simulink model "TosAeb"  (R2021a)
settings: Solver=FixedStepDiscrete  StartTime=0.0  StopTime=inf  FixedStep=0.01  RelTol=1e-3
undefined symbols (not defined in the file or in its folder): ACCModSts, AccSts1, AEB_ObjectMovingOrientation, AEB_ObjectMovingState, CN_BTCActive, CN_CurrentGearR, CN_CurrentGearR_DHT, CN_PTCActive, CN_VDCActive, CN_VSC_EspOff, CN_VSC_FCW_AEB_Error, CN_VSC_FCW_AEB_NoDispay, CN_VSC_FCW_AEB_PerfmDgdtn, CN_VSC_FCW_AEB_TempUnAvlbl, CN_VSC_VehStandstill, CNaccelAvoidMax, CNaccelAvoidMin, CNbrakePedalPress, CNcomfortStrLeftAccelMax, CNcomfortStrLeftAccelMin, CNcomfortStrRightAccelMax, CNcomfortStrRightAccelMin, CNDrvDoorOpen, CNDrvModeEco, CNDrvModeNorm, CNDrvModeOther, CNDrvModeSport, CNDrvSBRreminder, CNNull, CNobjInvalidID, CNPassDoorOpen, CNPassSBRreminder, CNPEBSAccelNegInf, CNPEBSAccelNull, CNPEBSTimeInf, CNPEBSTimeNull, CNSTOPMAX, CNSTOPNULL, CNsubjWidth, CNSwtOFF, CNSwtON, CNTTCMAX, CNTTCNULL, CNVehConfigAEBCycNone, CNVehConfigAEBNone, CNVehConfigAEBPedNone, CNVehConfigFCWNone, CNVehConfigJANone, CollisionSeverity, FALSE, FCWSnvty, JACycObjCalcInfoDefaultParams, JAPedObjCalcInfoDefaultParams, JAVehObjCalcInfoDefaultParams, latCycObjCalcInfoDefaultParams, latPedObjCalcInfoDefaultParams, longObjCalcInfoDefaultParams, ModSts, movingStatus, ObjectType ... (+1112)
libraries: simulink x3031, AEBSFunctionLib x50, Ta_Library x33, AEBSStateMachineLib x23, Mylibrary x12, simulink_extras x7, ObjSelect_lib x3, dspstat3 x2
(part 78 of 102)

== system TosAeb/FcwAebJa_Step_sys/ALG_AEBSystem/ALG_AEBController/AEB_LatObjInfoCalcApp/LatPedObjCalc/LatPedCollisonAssessInfo/LatPedCollisonAssessInfo/LatPedLongDistCondCalc/PedDistBrakeFullCalc/DistPhase3Calc/tPhase3Calc/tVelDeltaCalc ==
  velDelta : Inport  OutDataTypeStr=single
  subjLongAccelFilt : Inport  Port=2  OutDataTypeStr=single
  tJerkWork : Inport  Port=3  OutDataTypeStr=single
  Kjerk : Inport  Port=4  OutDataTypeStr=single
  accelMax : Inport  Port=5  OutDataTypeStr=single
  Add : Sum  Inputs=+--  OutDataTypeStr=single
  Constant : Constant  Value=0.5  OutDataTypeStr=single
  Divide : Product  Inputs=*/  OutDataTypeStr=single
  Product : Product  Inputs=4  OutDataTypeStr=single
  Product1 : Product  OutDataTypeStr=single
  tVelDelta : Outport
  wires:
  Divide/1 -> tVelDelta/1
  accelMax/1 -> Divide/2
  Kjerk/1 -> Product/3
  velDelta/1 -> Add/1
  Add/1 -> Divide/1
  Product1/1 -> Add/2
  Constant/1 -> Product/4
  tJerkWork/1 -> Product/2, Product/1, Product1/2
  Product/1 -> Add/3
  subjLongAccelFilt/1 -> Product1/1

== system TosAeb/FcwAebJa_Step_sys/ALG_AEBSystem/ALG_AEBController/AEB_LatObjInfoCalcApp/LatPedObjCalc/LatPedObjCalc/latPedObjInfoCalc/ObjColiisionRiskProbCalc/CollisionRiskProbCalc/ObjReginCorrCalc/AccelXYMaxMinCorrCalc/AccelXMaxMinCorrCalc ==
  vxAbsTargetCorr : Inport
  AccelMaxX : Inport  Port=2  OutDataTypeStr=single
  AccelMinX : Inport  Port=3  OutDataTypeStr=single
  Bus Creator : BusCreator  Inputs=2
  Bus Creator1 : BusCreator  Inputs=2
  Bus Creator2 : BusCreator  Inputs=2
  If : If  IfExpression=u1 < 0  ElseIfExpressions=u1 > 0
  Merge : Merge  Inputs=3
  ObjDwn2UpMoving : Subsystem  TreatAsAtomicUnit=on
  ObjStanding : Subsystem  TreatAsAtomicUnit=on
  ObjUp2DwnMoving : Subsystem  TreatAsAtomicUnit=on
  AccelXMaxMinCorr : Outport
  wires:
  [AccelMinX] -> ObjDwn2UpMoving/2(AccelMinX)
  vxAbsTargetCorr/1 -> If/1
  If/1 -> ObjUp2DwnMoving/Action
  If/2 -> [ObjDwn2Up]
  If/3 -> [ObjStand]
  [ObjDwn2Up] -> ObjDwn2UpMoving/Action
  [ObjStand] -> ObjStanding/Action
  AccelMinX/1 -> ObjUp2DwnMoving/2(AccelMinX), [AccelMinX]
  AccelMaxX/1 -> ObjUp2DwnMoving/1(AccelMaxX), ObjDwn2UpMoving/1(AccelMaxX)
  ObjUp2DwnMoving/1(AccelXMaxCorr) -> Bus Creator/1  "AccelXMaxCorr"
  ObjUp2DwnMoving/2(AccelXMinCorr) -> Bus Creator/2  "AccelXMinCorr"
  ObjDwn2UpMoving/1(AccelXMaxCorr) -> Bus Creator1/1  "AccelXMaxCorr"
  ObjDwn2UpMoving/2(AccelXMinCorr) -> Bus Creator1/2  "AccelXMinCorr"
  ObjStanding/1(AccelXMaxCorr) -> Bus Creator2/1  "AccelXMaxCorr"
  ObjStanding/2(AccelXMinCorr) -> Bus Creator2/2  "AccelXMinCorr"
  Bus Creator1/1 -> Merge/2
  Bus Creator/1 -> Merge/1
  Bus Creator2/1 -> Merge/3
  Merge/1 -> AccelXMaxMinCorr/1  "AccelXMaxMinCorr"

== system TosAeb/FcwAebJa_Step_sys/ALG_AEBSystem/ALG_AEBController/AEB_LatObjInfoCalcApp/LatPedObjCalc/LatPedObjCalc/latPedObjInfoCalc/ObjColiisionRiskProbCalc/CollisionRiskProbCalc/ObjReginCorrCalc/AccelXYMaxMinCorrCalc/AccelXYMaxMinCalc ==
  maxAccelDecel : Inport
  sincosCoef : Inport  Port=2
  Abs{1..4} (4 alike) : Abs
  Bus Creator : BusCreator  Inputs=2
  Bus Selector : BusSelector  OutputSignals=maxAccelDecel.maxAccel
  Bus Selector1 : BusSelector  OutputSignals=sincosCoef.cosCoef
  Bus Selector2 : BusSelector  OutputSignals=maxAccelDecel.maxDecel
  Bus Selector3 : BusSelector  OutputSignals=sincosCoef.cosCoef
  Bus Selector4 : BusSelector  OutputSignals=maxAccelDecel.maxAccel
  Bus Selector5 : BusSelector  OutputSignals=sincosCoef.sinCoef
  Bus Selector6 : BusSelector  OutputSignals=maxAccelDecel.maxDecel
  Bus Selector7 : BusSelector  OutputSignals=sincosCoef.sinCoef
  Product{2,4..6} (4 alike) : Product  OutDataTypeStr=single
  AccelXMax : Outport
  AccelXMin : Outport  Port=2
  AccelYMax : Outport  Port=3
  AccelYMin : Outport  Port=4
  wires:
  maxAccelDecel/1 -> Bus Creator/1
  sincosCoef/1 -> Bus Creator/2
  Bus Selector5/1 -> Abs1/1  "<sinCoef>"
  Bus Selector1/1 -> Abs4/1  "<cosCoef>"
  Bus Selector3/1 -> Abs3/1  "<cosCoef>"
  Bus Selector/1 -> Product2/1  "<maxAccel>"
  Product6/1 -> AccelYMin/1  "AccelYMin"
  Bus Selector4/1 -> Product5/1  "<maxAccel>"
  Bus Selector2/1 -> Product4/1  "<maxDecel>"
  Product4/1 -> AccelXMin/1  "AccelXMin"
  Bus Creator/1 -> Bus Selector7/1, Bus Selector6/1, Bus Selector5/1, Bus Selector4/1, Bus Selector3/1, Bus Selector2/1, Bus Selector1/1, Bus Selector/1
  Bus Selector7/1 -> Abs2/1  "<sinCoef>"
  Bus Selector6/1 -> Product6/1  "<maxDecel>"
  Product5/1 -> AccelYMax/1  "AccelYMax"
  Product2/1 -> AccelXMax/1  "AccelXMax"
  Abs2/1 -> Product6/2
  Abs1/1 -> Product5/2
  Abs3/1 -> Product4/2
  Abs4/1 -> Product2/2

== system TosAeb/FcwAebJa_Step_sys/ALG_AEBSystem/ALG_AEBController/AEB_LatObjInfoCalcApp/LatPedObjCalc/LatPedObjCalc/latPedObjInfoCalc/ObjColiisionRiskProbCalc/CollisionRiskProbCalc/ObjReginCorrCalc/AccelXYMaxMinCorrCalc/AccelYMaxMinCorrCalc ==
  vyAbsTargetCorr : Inport
  AccelMaxY : Inport  Port=2  OutDataTypeStr=single
  AccelMinY : Inport  Port=3  OutDataTypeStr=single
  Bus Creator : BusCreator  Inputs=2
  Bus Creator1 : BusCreator  Inputs=2
  Bus Creator2 : BusCreator  Inputs=2
  If : If  IfExpression=u1 < 0  ElseIfExpressions=u1 > 0
  Merge : Merge  Inputs=3
  ObjLeft2RightMoving : Subsystem  TreatAsAtomicUnit=on
  ObjRight2LeftMoving : Subsystem  TreatAsAtomicUnit=on
  ObjStanding : Subsystem  TreatAsAtomicUnit=on
  AccelYMaxMinCorr : Outport
  wires:
  Merge/1 -> AccelYMaxMinCorr/1  "AccelYMaxMinCorr"
  Bus Creator2/1 -> Merge/3
  Bus Creator/1 -> Merge/1
  Bus Creator1/1 -> Merge/2
  ObjStanding/2(AccelYMinCorr) -> Bus Creator2/2  "AccelYMinCorr"
  ObjStanding/1(AccelYMaxCorr) -> Bus Creator2/1  "AccelYMaxCorr"
  ObjRight2LeftMoving/2(AccelYMinCorr) -> Bus Creator1/2  "AccelYMinCorr"
  ObjRight2LeftMoving/1(AccelYMaxCorr) -> Bus Creator1/1  "AccelYMaxCorr"
  ObjLeft2RightMoving/2(AccelYMinCorr) -> Bus Creator/2  "AccelYMinCorr"
  ObjLeft2RightMoving/1(AccelYMaxCorr) -> Bus Creator/1  "AccelYMaxCorr"
  AccelMaxY/1 -> ObjRight2LeftMoving/1(AccelYMax), ObjLeft2RightMoving/1(AccelYMax)
  AccelMinY/1 -> [AccelMinX], ObjLeft2RightMoving/2(AccelYMin)
  [ObjStand] -> ObjStanding/Action
  [ObjRight2Left] -> ObjRight2LeftMoving/Action
  If/3 -> [ObjStand]
  If/2 -> [ObjRight2Left]
  If/1 -> ObjLeft2RightMoving/Action
  vyAbsTargetCorr/1 -> If/1
  [AccelMinX] -> ObjRight2LeftMoving/2(AccelYMin)

== system TosAeb/FcwAebJa_Step_sys/ALG_AEBSystem/ALG_AEBController/AEB_LatObjInfoCalcApp/LatPedObjCalc/LatPedObjCalc/latPedObjInfoCalc/ObjColiisionRiskProbCalc/CollisionRiskProbCalc/ObjReginCorrCalc/AccelXYMaxMinCorrCalc/sincosCoefCalc ==
  angle : Inport
  Bus Creator : BusCreator  Inputs=2
  Sincos : Trigonometry  Operator=sincos
  sincosCoef : Outport
  wires:
  Sincos/1 -> Bus Creator/1  "sinCoef"
  angle/1 -> Sincos/1
  Sincos/2 -> Bus Creator/2  "cosCoef"
  Bus Creator/1 -> sincosCoef/1  "sincosCoef"

== system TosAeb/FcwAebJa_Step_sys/ALG_AEBSystem/ALG_AEBController/AEB_LatObjInfoCalcApp/LatPedObjCalc/LatPedObjCalc/latPedObjInfoCalc/ObjColiisionRiskProbCalc/CollisionRiskProbCalc/ObjReginCorrCalc/AccelXYMaxMinCorrCalc/vXYAngleCalc ==
  note: vy
      | vxAngle = atan(--------)
      | 				vx
  velAbsTargetCorr : Inport
  Bus Selector : BusSelector  OutputSignals=vyAbsObjCorr,vxAbsObjCorr
  Trigonometric Function : Trigonometry  Operator=atan2
  vXYAngle : Outport
  wires:
  velAbsTargetCorr/1 -> Bus Selector/1
  Trigonometric Function/1 -> vXYAngle/1
  Bus Selector/1 -> Trigonometric Function/1  "<vyAbsObjCorr>"
  Bus Selector/2 -> Trigonometric Function/2  "<vxAbsObjCorr>"

== system TosAeb/FcwAebJa_Step_sys/ALG_AEBSystem/ALG_AEBController/AEB_LatObjInfoCalcApp/LatPedObjCalc/LatPedObjCalc/latPedObjInfoCalc/ObjColiisionRiskProbCalc/CollisionRiskProbCalc/ObjReginCorrCalc/PedMaxAccelCalc/MaxAccelJoggingMode ==
  tPredTime : Inport  OutDataTypeStr=single
  Action Port : ActionPort  ActionPortLabel=case [ 2 ]:
  Bus Creator : BusCreator  Inputs=2
  MaxAccelJoggingModeLookupTable : Lookup_n-D  NumberOfTableDimensions=1  BreakpointsForDimension1=P_AEB_LatPedCA_CollisionRiskProbJoggingStsMaxAccelX  BeginIndexSearchUsingPreviousIndexResult=on  Table=P_AEB_LatPedCA_CollisionRiskProbJoggingStsMaxAccelY  ExtrapMethod=Clip  InternalRulePriority=Speed  OutDataTypeStr=single  RndMeth=Simplest  InputSameDT=on
  MaxDecelJoggingModeLookupTable1 : Lookup_n-D  NumberOfTableDimensions=1  BreakpointsForDimension1=P_AEB_LatPedCA_CollisionRiskProbJoggingStsMaxDecelX  BeginIndexSearchUsingPreviousIndexResult=on  Table=P_AEB_LatPedCA_CollisionRiskProbJoggingStsMaxDecelY  ExtrapMethod=Clip  InternalRulePriority=Speed  OutDataTypeStr=single  RndMeth=Simplest  InputSameDT=on
  maxAccelDecel : Outport
  wires:
  tPredTime/1 -> MaxDecelJoggingModeLookupTable1/1, MaxAccelJoggingModeLookupTable/1
  MaxAccelJoggingModeLookupTable/1 -> Bus Creator/1  "maxAccel"
  Bus Creator/1 -> maxAccelDecel/1
  MaxDecelJoggingModeLookupTable1/1 -> Bus Creator/2  "maxDecel"

== system TosAeb/FcwAebJa_Step_sys/ALG_AEBSystem/ALG_AEBController/AEB_LatObjInfoCalcApp/LatPedObjCalc/LatPedObjCalc/latPedObjInfoCalc/ObjColiisionRiskProbCalc/CollisionRiskProbCalc/ObjReginCorrCalc/PedMaxAccelCalc/MaxAccelRunningMode ==
  tPredTime : Inport  OutDataTypeStr=single
  Action Port : ActionPort  ActionPortLabel=default:
  Bus Creator : BusCreator  Inputs=2
  MaxAccelRunningModeLookupTable : Lookup_n-D  NumberOfTableDimensions=1  BreakpointsForDimension1=P_AEB_LatPedCA_CollisionRiskProbRunningStsMaxAccelX  BeginIndexSearchUsingPreviousIndexResult=on  Table=P_AEB_LatPedCA_CollisionRiskProbRunningStsMaxAccelY  ExtrapMethod=Clip  InternalRulePriority=Speed  OutDataTypeStr=single  RndMeth=Simplest  InputSameDT=on
  MaxDecelRuningModeLookupTable : Lookup_n-D  NumberOfTableDimensions=1  BreakpointsForDimension1=P_AEB_LatPedCA_CollisionRiskProbRunningStsMaxDecelX  BeginIndexSearchUsingPreviousIndexResult=on  Table=P_AEB_LatPedCA_CollisionRiskProbRunningStsMaxDecelY  ExtrapMethod=Clip  InternalRulePriority=Speed  OutDataTypeStr=single  RndMeth=Simplest  InputSameDT=on
  maxAccelDecel : Outport
  wires:
  tPredTime/1 -> MaxDecelRuningModeLookupTable/1, MaxAccelRunningModeLookupTable/1
  MaxAccelRunningModeLookupTable/1 -> Bus Creator/1  "maxAccel"
  Bus Creator/1 -> maxAccelDecel/1
  MaxDecelRuningModeLookupTable/1 -> Bus Creator/2  "maxDecel"

== system TosAeb/FcwAebJa_Step_sys/ALG_AEBSystem/ALG_AEBController/AEB_LatObjInfoCalcApp/LatPedObjCalc/LatPedObjCalc/latPedObjInfoCalc/ObjColiisionRiskProbCalc/CollisionRiskProbCalc/ObjReginCorrCalc/PedMaxAccelCalc/MaxAccelStoppedMode ==
  tPredTime : Inport  OutDataTypeStr=single
  Action Port : ActionPort  ActionPortLabel=case [ 0 ]:
  Bus Creator : BusCreator  Inputs=2
  MaxAccelStoppedModeLookupTable : Lookup_n-D  NumberOfTableDimensions=1  BreakpointsForDimension1=P_AEB_LatPedCA_CollisionRiskProbStopStsMaxAccelX  BeginIndexSearchUsingPreviousIndexResult=on  Table=P_AEB_LatPedCA_CollisionRiskProbStopStsMaxAccelY  ExtrapMethod=Clip  InternalRulePriority=Speed  OutDataTypeStr=single  RndMeth=Simplest  InputSameDT=on
  MaxDecelStoppedModeLookupTable1 : Lookup_n-D  NumberOfTableDimensions=1  BreakpointsForDimension1=P_AEB_LatPedCA_CollisionRiskProbStopStsMaxDecelX  BeginIndexSearchUsingPreviousIndexResult=on  Table=P_AEB_LatPedCA_CollisionRiskProbStopStsMaxDecelY  ExtrapMethod=Clip  InternalRulePriority=Speed  OutDataTypeStr=single  RndMeth=Simplest  InputSameDT=on
  maxAccelDecel : Outport
  wires:
  MaxAccelStoppedModeLookupTable/1 -> Bus Creator/1  "maxAccel"
  tPredTime/1 -> MaxDecelStoppedModeLookupTable1/1, MaxAccelStoppedModeLookupTable/1
  Bus Creator/1 -> maxAccelDecel/1
  MaxDecelStoppedModeLookupTable1/1 -> Bus Creator/2  "maxDecel"

== system TosAeb/FcwAebJa_Step_sys/ALG_AEBSystem/ALG_AEBController/AEB_LatObjInfoCalcApp/LatPedObjCalc/LatPedObjCalc/latPedObjInfoCalc/ObjColiisionRiskProbCalc/CollisionRiskProbCalc/ObjReginCorrCalc/PedMaxAccelCalc/MaxAccelWalkingMode ==
  tPredTime : Inport  OutDataTypeStr=single
  Action Port : ActionPort  ActionPortLabel=case [ 1 ]:
  Bus Creator : BusCreator  Inputs=2
  MaxAccelWalkingModeLookupTable : Lookup_n-D  NumberOfTableDimensions=1  BreakpointsForDimension1=P_AEB_LatPedCA_CollisionRiskProbWalkingStsMaxAccelX  BeginIndexSearchUsingPreviousIndexResult=on  Table=P_AEB_LatPedCA_CollisionRiskProbWalkingStsMaxAccelY  ExtrapMethod=Clip  InternalRulePriority=Speed  OutDataTypeStr=single  RndMeth=Simplest  InputSameDT=on
  MaxDecelWalkingModeLookupTable1 : Lookup_n-D  NumberOfTableDimensions=1  BreakpointsForDimension1=P_AEB_LatPedCA_CollisionRiskProbWalkingStsMaxDecelX  BeginIndexSearchUsingPreviousIndexResult=on  Table=P_AEB_LatPedCA_CollisionRiskProbWalkingStsMaxDecelY  ExtrapMethod=Clip  InternalRulePriority=Speed  OutDataTypeStr=single  RndMeth=Simplest  InputSameDT=on
  maxAccelDecel : Outport
  wires:
  tPredTime/1 -> MaxDecelWalkingModeLookupTable1/1, MaxAccelWalkingModeLookupTable/1
  MaxAccelWalkingModeLookupTable/1 -> Bus Creator/1  "maxAccel"
  Bus Creator/1 -> maxAccelDecel/1
  MaxDecelWalkingModeLookupTable1/1 -> Bus Creator/2  "maxDecel"

== system TosAeb/FcwAebJa_Step_sys/ALG_AEBSystem/ALG_AEBController/AEB_LatObjInfoCalcApp/LatPedObjCalc/LatPedObjCalc/latPedObjInfoCalc/ObjCrossFlgCal/PedPreCollisionCalcFunc/PreCrossFlgCalcFlow/VectorCrossAlgorithm/If Action Subsystem1/Subsystem ==
  temp_u : Inport  OutDataTypeStr=single
  temp_t : Inport  Port=2  OutDataTypeStr=single
  vectorMulit : Inport  Port=3
  Compare To Constant : simulink/Logic and Bit Operations/Compare To Constant  relop=>=  const=0  OutDataTypeStr=boolean
  Compare To Constant1 : simulink/Logic and Bit Operations/Compare To Constant  relop=<=  const=1  OutDataTypeStr=boolean
  Compare To Constant2 : simulink/Logic and Bit Operations/Compare To Constant  relop=>=  const=0  OutDataTypeStr=boolean
  Compare To Constant3 : simulink/Logic and Bit Operations/Compare To Constant  relop=<=  const=1  OutDataTypeStr=boolean
  Divide : Product  Inputs=*/
  Divide1 : Product  Inputs=*/
  Logical Operator : Logic  Inputs=4  AllPortsSameDT=off  OutDataTypeStr=boolean
  CrossFlg : Outport
  wires:
  vectorMulit/1 -> Divide1/2, Divide/2
  temp_t/1 -> Divide/1
  temp_u/1 -> Divide1/1
  Divide1/1 -> Compare To Constant1/1, Compare To Constant/1
  Divide/1 -> Compare To Constant3/1, Compare To Constant2/1
  Compare To Constant1/1 -> Logical Operator/2
  Compare To Constant2/1 -> Logical Operator/3
  Compare To Constant/1 -> Logical Operator/1
  Compare To Constant3/1 -> Logical Operator/4
  Logical Operator/1 -> CrossFlg/1

== system TosAeb/FcwAebJa_Step_sys/ALG_AEBSystem/ALG_AEBController/AEB_LatObjInfoCalcApp/LatPedObjCalc/LatPedObjCalc/latPedObjInfoCalc/ObjCrossFlgCal/PedPreCollisionCalcFunc/PreCrossFlgCalcFlow/VectorCrossAlgorithm/If Action Subsystem1/t ==
  m2 : Inport  OutDataTypeStr=single
  m1 : Inport  Port=2  OutDataTypeStr=single
  y1 : Inport  Port=3  OutDataTypeStr=single
  n1 : Inport  Port=4  OutDataTypeStr=single
  n2 : Inport  Port=5  OutDataTypeStr=single
  x1 : Inport  Port=6  OutDataTypeStr=single
  Product : Product  OutDataTypeStr=single
  Product1 : Product  OutDataTypeStr=single
  Subtract{1..5} (5 alike) : Sum  Inputs=+-  OutDataTypeStr=single
  temp_t : Outport
  wires:
  m2/1 -> Subtract2/1
  m1/1 -> Subtract2/2, Subtract1/2
  y1/1 -> Subtract3/1
  Subtract2/1 -> Product/1
  Subtract3/1 -> Product/2
  n2/1 -> Subtract4/1
  n1/1 -> Subtract4/2, Subtract3/2
  x1/1 -> Subtract1/1
  Subtract4/1 -> Product1/1
  Subtract1/1 -> Product1/2
  Product/1 -> Subtract5/1
  Product1/1 -> Subtract5/2
  Subtract5/1 -> temp_t/1

== system TosAeb/FcwAebJa_Step_sys/ALG_AEBSystem/ALG_AEBController/AEB_LatObjInfoCalcApp/LatPedObjCalc/LatPedObjCalc/latPedObjInfoCalc/ObjCrossFlgCal/PedPreCollisionCalcFunc/PreCrossFlgCalcFlow/VectorCrossAlgorithm/If Action Subsystem1/u ==
  x2 : Inport  OutDataTypeStr=single
  x1 : Inport  Port=2  OutDataTypeStr=single
  y1 : Inport  Port=3  OutDataTypeStr=single
  n1 : Inport  Port=4  OutDataTypeStr=single
  y2 : Inport  Port=5  OutDataTypeStr=single
  m1 : Inport  Port=6  OutDataTypeStr=single
  Product : Product  OutDataTypeStr=single
  Product1 : Product  OutDataTypeStr=single
  Subtract{1..5} (5 alike) : Sum  Inputs=+-  OutDataTypeStr=single
  temp_u : Outport
  wires:
  Product1/1 -> Subtract5/2
  Product/1 -> Subtract5/1
  Subtract1/1 -> Product1/2
  Subtract4/1 -> Product1/1
  n1/1 -> Subtract3/2
  y2/1 -> Subtract4/1
  Subtract3/1 -> Product/2
  Subtract2/1 -> Product/1
  y1/1 -> Subtract4/2, Subtract3/1
  x1/1 -> Subtract1/1, Subtract2/2
  x2/1 -> Subtract2/1
  m1/1 -> Subtract1/2
  Subtract5/1 -> temp_u/1

== system TosAeb/FcwAebJa_Step_sys/ALG_AEBSystem/ALG_AEBController/AEB_LatObjInfoCalcApp/LatPedObjCalc/Subsystem/latPedObjInfoCalc/ObjColiisionRiskProbCalc/CollisionRiskProbCalc/ObjReginCorrCalc/AccelXYMaxMinCorrCalc/AccelXMaxMinCorrCalc ==
  vxAbsTargetCorr : Inport
  AccelMaxX : Inport  Port=2  OutDataTypeStr=single
  AccelMinX : Inport  Port=3  OutDataTypeStr=single
  Bus Creator : BusCreator  Inputs=2
  Bus Creator1 : BusCreator  Inputs=2
  Bus Creator2 : BusCreator  Inputs=2
  If : If  IfExpression=u1 < 0  ElseIfExpressions=u1 > 0
  Merge : Merge  Inputs=3
  ObjDwn2UpMoving : Subsystem  TreatAsAtomicUnit=on
  ObjStanding : Subsystem  TreatAsAtomicUnit=on
  ObjUp2DwnMoving : Subsystem  TreatAsAtomicUnit=on
  AccelXMaxMinCorr : Outport
  wires:
  [AccelMinX] -> ObjDwn2UpMoving/2(AccelMinX)
  vxAbsTargetCorr/1 -> If/1
  If/1 -> ObjUp2DwnMoving/Action
  If/2 -> [ObjDwn2Up]
  If/3 -> [ObjStand]
  [ObjDwn2Up] -> ObjDwn2UpMoving/Action
  [ObjStand] -> ObjStanding/Action
  AccelMinX/1 -> ObjUp2DwnMoving/2(AccelMinX), [AccelMinX]
  AccelMaxX/1 -> ObjUp2DwnMoving/1(AccelMaxX), ObjDwn2UpMoving/1(AccelMaxX)
  ObjUp2DwnMoving/1(AccelXMaxCorr) -> Bus Creator/1  "AccelXMaxCorr"
  ObjUp2DwnMoving/2(AccelXMinCorr) -> Bus Creator/2  "AccelXMinCorr"
  ObjDwn2UpMoving/1(AccelXMaxCorr) -> Bus Creator1/1  "AccelXMaxCorr"
  ObjDwn2UpMoving/2(AccelXMinCorr) -> Bus Creator1/2  "AccelXMinCorr"
  ObjStanding/1(AccelXMaxCorr) -> Bus Creator2/1  "AccelXMaxCorr"
  ObjStanding/2(AccelXMinCorr) -> Bus Creator2/2  "AccelXMinCorr"
  Bus Creator1/1 -> Merge/2
  Bus Creator/1 -> Merge/1
  Bus Creator2/1 -> Merge/3
  Merge/1 -> AccelXMaxMinCorr/1  "AccelXMaxMinCorr"

== system TosAeb/FcwAebJa_Step_sys/ALG_AEBSystem/ALG_AEBController/AEB_LatObjInfoCalcApp/LatPedObjCalc/Subsystem/latPedObjInfoCalc/ObjColiisionRiskProbCalc/CollisionRiskProbCalc/ObjReginCorrCalc/AccelXYMaxMinCorrCalc/AccelXYMaxMinCalc ==
  maxAccelDecel : Inport
  sincosCoef : Inport  Port=2
  Abs{1..4} (4 alike) : Abs
  Bus Creator : BusCreator  Inputs=2
  Bus Selector : BusSelector  OutputSignals=maxAccelDecel.maxAccel
  Bus Selector1 : BusSelector  OutputSignals=sincosCoef.cosCoef
  Bus Selector2 : BusSelector  OutputSignals=maxAccelDecel.maxDecel
  Bus Selector3 : BusSelector  OutputSignals=sincosCoef.cosCoef
  Bus Selector4 : BusSelector  OutputSignals=maxAccelDecel.maxAccel
  Bus Selector5 : BusSelector  OutputSignals=sincosCoef.sinCoef
  Bus Selector6 : BusSelector  OutputSignals=maxAccelDecel.maxDecel
  Bus Selector7 : BusSelector  OutputSignals=sincosCoef.sinCoef
  Product{2,4..6} (4 alike) : Product  OutDataTypeStr=single
  AccelXMax : Outport
  AccelXMin : Outport  Port=2
  AccelYMax : Outport  Port=3
  AccelYMin : Outport  Port=4
  wires:
  maxAccelDecel/1 -> Bus Creator/1
  sincosCoef/1 -> Bus Creator/2
  Bus Selector5/1 -> Abs1/1  "<sinCoef>"
  Bus Selector1/1 -> Abs4/1  "<cosCoef>"
  Bus Selector3/1 -> Abs3/1  "<cosCoef>"
  Bus Selector/1 -> Product2/1  "<maxAccel>"
  Product6/1 -> AccelYMin/1  "AccelYMin"
  Bus Selector4/1 -> Product5/1  "<maxAccel>"
  Bus Selector2/1 -> Product4/1  "<maxDecel>"
  Product4/1 -> AccelXMin/1  "AccelXMin"
  Bus Creator/1 -> Bus Selector7/1, Bus Selector6/1, Bus Selector5/1, Bus Selector4/1, Bus Selector3/1, Bus Selector2/1, Bus Selector1/1, Bus Selector/1
  Bus Selector7/1 -> Abs2/1  "<sinCoef>"
  Bus Selector6/1 -> Product6/1  "<maxDecel>"
  Product5/1 -> AccelYMax/1  "AccelYMax"
  Product2/1 -> AccelXMax/1  "AccelXMax"
  Abs2/1 -> Product6/2
  Abs1/1 -> Product5/2
  Abs3/1 -> Product4/2
  Abs4/1 -> Product2/2

== system TosAeb/FcwAebJa_Step_sys/ALG_AEBSystem/ALG_AEBController/AEB_LatObjInfoCalcApp/LatPedObjCalc/Subsystem/latPedObjInfoCalc/ObjColiisionRiskProbCalc/CollisionRiskProbCalc/ObjReginCorrCalc/AccelXYMaxMinCorrCalc/AccelYMaxMinCorrCalc ==
  vyAbsTargetCorr : Inport
  AccelMaxY : Inport  Port=2  OutDataTypeStr=single
  AccelMinY : Inport  Port=3  OutDataTypeStr=single
  Bus Creator : BusCreator  Inputs=2
  Bus Creator1 : BusCreator  Inputs=2
  Bus Creator2 : BusCreator  Inputs=2
  If : If  IfExpression=u1 < 0  ElseIfExpressions=u1 > 0
  Merge : Merge  Inputs=3
  ObjLeft2RightMoving : Subsystem  TreatAsAtomicUnit=on
  ObjRight2LeftMoving : Subsystem  TreatAsAtomicUnit=on
  ObjStanding : Subsystem  TreatAsAtomicUnit=on
  AccelYMaxMinCorr : Outport
  wires:
  Merge/1 -> AccelYMaxMinCorr/1  "AccelYMaxMinCorr"
  Bus Creator2/1 -> Merge/3
  Bus Creator/1 -> Merge/1
  Bus Creator1/1 -> Merge/2
  ObjStanding/2(AccelYMinCorr) -> Bus Creator2/2  "AccelYMinCorr"
  ObjStanding/1(AccelYMaxCorr) -> Bus Creator2/1  "AccelYMaxCorr"
  ObjRight2LeftMoving/2(AccelYMinCorr) -> Bus Creator1/2  "AccelYMinCorr"
  ObjRight2LeftMoving/1(AccelYMaxCorr) -> Bus Creator1/1  "AccelYMaxCorr"
  ObjLeft2RightMoving/2(AccelYMinCorr) -> Bus Creator/2  "AccelYMinCorr"
  ObjLeft2RightMoving/1(AccelYMaxCorr) -> Bus Creator/1  "AccelYMaxCorr"
  AccelMaxY/1 -> ObjRight2LeftMoving/1(AccelYMax), ObjLeft2RightMoving/1(AccelYMax)
  AccelMinY/1 -> [AccelMinX], ObjLeft2RightMoving/2(AccelYMin)
  [ObjStand] -> ObjStanding/Action
  [ObjRight2Left] -> ObjRight2LeftMoving/Action
  If/3 -> [ObjStand]
  If/2 -> [ObjRight2Left]
  If/1 -> ObjLeft2RightMoving/Action
  vyAbsTargetCorr/1 -> If/1
  [AccelMinX] -> ObjRight2LeftMoving/2(AccelYMin)

== system TosAeb/FcwAebJa_Step_sys/ALG_AEBSystem/ALG_AEBController/AEB_LatObjInfoCalcApp/LatPedObjCalc/Subsystem/latPedObjInfoCalc/ObjColiisionRiskProbCalc/CollisionRiskProbCalc/ObjReginCorrCalc/AccelXYMaxMinCorrCalc/sincosCoefCalc ==
  angle : Inport
  Bus Creator : BusCreator  Inputs=2
  Sincos : Trigonometry  Operator=sincos
  sincosCoef : Outport
  wires:
  Sincos/1 -> Bus Creator/1  "sinCoef"
  angle/1 -> Sincos/1
  Sincos/2 -> Bus Creator/2  "cosCoef"
  Bus Creator/1 -> sincosCoef/1  "sincosCoef"

== system TosAeb/FcwAebJa_Step_sys/ALG_AEBSystem/ALG_AEBController/AEB_LatObjInfoCalcApp/LatPedObjCalc/Subsystem/latPedObjInfoCalc/ObjColiisionRiskProbCalc/CollisionRiskProbCalc/ObjReginCorrCalc/AccelXYMaxMinCorrCalc/vXYAngleCalc ==
  note: vy
      | vxAngle = atan(--------)
      | 				vx
  velAbsTargetCorr : Inport
  Bus Selector : BusSelector  OutputSignals=vyAbsObjCorr,vxAbsObjCorr
  Trigonometric Function : Trigonometry  Operator=atan2
  vXYAngle : Outport
  wires:
  velAbsTargetCorr/1 -> Bus Selector/1
  Trigonometric Function/1 -> vXYAngle/1
  Bus Selector/1 -> Trigonometric Function/1  "<vyAbsObjCorr>"
  Bus Selector/2 -> Trigonometric Function/2  "<vxAbsObjCorr>"

== system TosAeb/FcwAebJa_Step_sys/ALG_AEBSystem/ALG_AEBController/AEB_LatObjInfoCalcApp/LatPedObjCalc/Subsystem/latPedObjInfoCalc/ObjColiisionRiskProbCalc/CollisionRiskProbCalc/ObjReginCorrCalc/PedMaxAccelCalc/MaxAccelJoggingMode ==
  tPredTime : Inport  OutDataTypeStr=single
  Action Port : ActionPort  ActionPortLabel=case [ 2 ]:
  Bus Creator : BusCreator  Inputs=2
  MaxAccelJoggingModeLookupTable : Lookup_n-D  NumberOfTableDimensions=1  BreakpointsForDimension1=P_AEB_LatPedCA_CollisionRiskProbJoggingStsMaxAccelX  BeginIndexSearchUsingPreviousIndexResult=on  Table=P_AEB_LatPedCA_CollisionRiskProbJoggingStsMaxAccelY  ExtrapMethod=Clip  InternalRulePriority=Speed  OutDataTypeStr=single  RndMeth=Simplest  InputSameDT=on
  MaxDecelJoggingModeLookupTable1 : Lookup_n-D  NumberOfTableDimensions=1  BreakpointsForDimension1=P_AEB_LatPedCA_CollisionRiskProbJoggingStsMaxDecelX  BeginIndexSearchUsingPreviousIndexResult=on  Table=P_AEB_LatPedCA_CollisionRiskProbJoggingStsMaxDecelY  ExtrapMethod=Clip  InternalRulePriority=Speed  OutDataTypeStr=single  RndMeth=Simplest  InputSameDT=on
  maxAccelDecel : Outport
  wires:
  tPredTime/1 -> MaxDecelJoggingModeLookupTable1/1, MaxAccelJoggingModeLookupTable/1
  MaxAccelJoggingModeLookupTable/1 -> Bus Creator/1  "maxAccel"
  Bus Creator/1 -> maxAccelDecel/1
  MaxDecelJoggingModeLookupTable1/1 -> Bus Creator/2  "maxDecel"

== system TosAeb/FcwAebJa_Step_sys/ALG_AEBSystem/ALG_AEBController/AEB_LatObjInfoCalcApp/LatPedObjCalc/Subsystem/latPedObjInfoCalc/ObjColiisionRiskProbCalc/CollisionRiskProbCalc/ObjReginCorrCalc/PedMaxAccelCalc/MaxAccelRunningMode ==
  tPredTime : Inport  OutDataTypeStr=single
  Action Port : ActionPort  ActionPortLabel=default:
  Bus Creator : BusCreator  Inputs=2
  MaxAccelRunningModeLookupTable : Lookup_n-D  NumberOfTableDimensions=1  BreakpointsForDimension1=P_AEB_LatPedCA_CollisionRiskProbRunningStsMaxAccelX  BeginIndexSearchUsingPreviousIndexResult=on  Table=P_AEB_LatPedCA_CollisionRiskProbRunningStsMaxAccelY  ExtrapMethod=Clip  InternalRulePriority=Speed  OutDataTypeStr=single  RndMeth=Simplest  InputSameDT=on
  MaxDecelRuningModeLookupTable : Lookup_n-D  NumberOfTableDimensions=1  BreakpointsForDimension1=P_AEB_LatPedCA_CollisionRiskProbRunningStsMaxDecelX  BeginIndexSearchUsingPreviousIndexResult=on  Table=P_AEB_LatPedCA_CollisionRiskProbRunningStsMaxDecelY  ExtrapMethod=Clip  InternalRulePriority=Speed  OutDataTypeStr=single  RndMeth=Simplest  InputSameDT=on
  maxAccelDecel : Outport
  wires:
  tPredTime/1 -> MaxDecelRuningModeLookupTable/1, MaxAccelRunningModeLookupTable/1
  MaxAccelRunningModeLookupTable/1 -> Bus Creator/1  "maxAccel"
  Bus Creator/1 -> maxAccelDecel/1
  MaxDecelRuningModeLookupTable/1 -> Bus Creator/2  "maxDecel"

== system TosAeb/FcwAebJa_Step_sys/ALG_AEBSystem/ALG_AEBController/AEB_LatObjInfoCalcApp/LatPedObjCalc/Subsystem/latPedObjInfoCalc/ObjColiisionRiskProbCalc/CollisionRiskProbCalc/ObjReginCorrCalc/PedMaxAccelCalc/MaxAccelStoppedMode ==
  tPredTime : Inport  OutDataTypeStr=single
  Action Port : ActionPort  ActionPortLabel=case [ 0 ]:
  Bus Creator : BusCreator  Inputs=2
  MaxAccelStoppedModeLookupTable : Lookup_n-D  NumberOfTableDimensions=1  BreakpointsForDimension1=P_AEB_LatPedCA_CollisionRiskProbStopStsMaxAccelX  BeginIndexSearchUsingPreviousIndexResult=on  Table=P_AEB_LatPedCA_CollisionRiskProbStopStsMaxAccelY  ExtrapMethod=Clip  InternalRulePriority=Speed  OutDataTypeStr=single  RndMeth=Simplest  InputSameDT=on
  MaxDecelStoppedModeLookupTable1 : Lookup_n-D  NumberOfTableDimensions=1  BreakpointsForDimension1=P_AEB_LatPedCA_CollisionRiskProbStopStsMaxDecelX  BeginIndexSearchUsingPreviousIndexResult=on  Table=P_AEB_LatPedCA_CollisionRiskProbStopStsMaxDecelY  ExtrapMethod=Clip  InternalRulePriority=Speed  OutDataTypeStr=single  RndMeth=Simplest  InputSameDT=on
  maxAccelDecel : Outport
  wires:
  MaxAccelStoppedModeLookupTable/1 -> Bus Creator/1  "maxAccel"
  tPredTime/1 -> MaxDecelStoppedModeLookupTable1/1, MaxAccelStoppedModeLookupTable/1
  Bus Creator/1 -> maxAccelDecel/1
  MaxDecelStoppedModeLookupTable1/1 -> Bus Creator/2  "maxDecel"

== system TosAeb/FcwAebJa_Step_sys/ALG_AEBSystem/ALG_AEBController/AEB_LatObjInfoCalcApp/LatPedObjCalc/Subsystem/latPedObjInfoCalc/ObjColiisionRiskProbCalc/CollisionRiskProbCalc/ObjReginCorrCalc/PedMaxAccelCalc/MaxAccelWalkingMode ==
  tPredTime : Inport  OutDataTypeStr=single
  Action Port : ActionPort  ActionPortLabel=case [ 1 ]:
  Bus Creator : BusCreator  Inputs=2
  MaxAccelWalkingModeLookupTable : Lookup_n-D  NumberOfTableDimensions=1  BreakpointsForDimension1=P_AEB_LatPedCA_CollisionRiskProbWalkingStsMaxAccelX  BeginIndexSearchUsingPreviousIndexResult=on  Table=P_AEB_LatPedCA_CollisionRiskProbWalkingStsMaxAccelY  ExtrapMethod=Clip  InternalRulePriority=Speed  OutDataTypeStr=single  RndMeth=Simplest  InputSameDT=on
  MaxDecelWalkingModeLookupTable1 : Lookup_n-D  NumberOfTableDimensions=1  BreakpointsForDimension1=P_AEB_LatPedCA_CollisionRiskProbWalkingStsMaxDecelX  BeginIndexSearchUsingPreviousIndexResult=on  Table=P_AEB_LatPedCA_CollisionRiskProbWalkingStsMaxDecelY  ExtrapMethod=Clip  InternalRulePriority=Speed  OutDataTypeStr=single  RndMeth=Simplest  InputSameDT=on
  maxAccelDecel : Outport
  wires:
  tPredTime/1 -> MaxDecelWalkingModeLookupTable1/1, MaxAccelWalkingModeLookupTable/1
  MaxAccelWalkingModeLookupTable/1 -> Bus Creator/1  "maxAccel"
  Bus Creator/1 -> maxAccelDecel/1
  MaxDecelWalkingModeLookupTable1/1 -> Bus Creator/2  "maxDecel"

== system TosAeb/FcwAebJa_Step_sys/ALG_AEBSystem/ALG_AEBController/AEB_LatObjInfoCalcApp/LatPedObjCalc/Subsystem/latPedObjInfoCalc/ObjCrossFlgCal/PedPreCollisionCalcFunc/PreCrossFlgCalcFlow/VectorCrossAlgorithm/If Action Subsystem1/Subsystem ==
  temp_u : Inport  OutDataTypeStr=single
  temp_t : Inport  Port=2  OutDataTypeStr=single
  vectorMulit : Inport  Port=3
  Compare To Constant : simulink/Logic and Bit Operations/Compare To Constant  relop=>=  const=0  OutDataTypeStr=boolean
  Compare To Constant1 : simulink/Logic and Bit Operations/Compare To Constant  relop=<=  const=1  OutDataTypeStr=boolean
  Compare To Constant2 : simulink/Logic and Bit Operations/Compare To Constant  relop=>=  const=0  OutDataTypeStr=boolean
  Compare To Constant3 : simulink/Logic and Bit Operations/Compare To Constant  relop=<=  const=1  OutDataTypeStr=boolean
  Divide : Product  Inputs=*/
  Divide1 : Product  Inputs=*/
  Logical Operator : Logic  Inputs=4  AllPortsSameDT=off  OutDataTypeStr=boolean
  CrossFlg : Outport
  wires:
  vectorMulit/1 -> Divide1/2, Divide/2
  temp_t/1 -> Divide/1
  temp_u/1 -> Divide1/1
  Divide1/1 -> Compare To Constant1/1, Compare To Constant/1
  Divide/1 -> Compare To Constant3/1, Compare To Constant2/1
  Compare To Constant1/1 -> Logical Operator/2
  Compare To Constant2/1 -> Logical Operator/3
  Compare To Constant/1 -> Logical Operator/1
  Compare To Constant3/1 -> Logical Operator/4
  Logical Operator/1 -> CrossFlg/1

== system TosAeb/FcwAebJa_Step_sys/ALG_AEBSystem/ALG_AEBController/AEB_LatObjInfoCalcApp/LatPedObjCalc/Subsystem/latPedObjInfoCalc/ObjCrossFlgCal/PedPreCollisionCalcFunc/PreCrossFlgCalcFlow/VectorCrossAlgorithm/If Action Subsystem1/t ==
  m2 : Inport  OutDataTypeStr=single
  m1 : Inport  Port=2  OutDataTypeStr=single
  y1 : Inport  Port=3  OutDataTypeStr=single
  n1 : Inport  Port=4  OutDataTypeStr=single
  n2 : Inport  Port=5  OutDataTypeStr=single
  x1 : Inport  Port=6  OutDataTypeStr=single
  Product : Product  OutDataTypeStr=single
  Product1 : Product  OutDataTypeStr=single
  Subtract{1..5} (5 alike) : Sum  Inputs=+-  OutDataTypeStr=single
  temp_t : Outport
  wires:
  m2/1 -> Subtract2/1
  m1/1 -> Subtract2/2, Subtract1/2
  y1/1 -> Subtract3/1
  Subtract2/1 -> Product/1
  Subtract3/1 -> Product/2
  n2/1 -> Subtract4/1
  n1/1 -> Subtract4/2, Subtract3/2
  x1/1 -> Subtract1/1
  Subtract4/1 -> Product1/1
  Subtract1/1 -> Product1/2
  Product/1 -> Subtract5/1
  Product1/1 -> Subtract5/2
  Subtract5/1 -> temp_t/1

== system TosAeb/FcwAebJa_Step_sys/ALG_AEBSystem/ALG_AEBController/AEB_LatObjInfoCalcApp/LatPedObjCalc/Subsystem/latPedObjInfoCalc/ObjCrossFlgCal/PedPreCollisionCalcFunc/PreCrossFlgCalcFlow/VectorCrossAlgorithm/If Action Subsystem1/u ==
  x2 : Inport  OutDataTypeStr=single
  x1 : Inport  Port=2  OutDataTypeStr=single
  y1 : Inport  Port=3  OutDataTypeStr=single
  n1 : Inport  Port=4  OutDataTypeStr=single
  y2 : Inport  Port=5  OutDataTypeStr=single
  m1 : Inport  Port=6  OutDataTypeStr=single
  Product : Product  OutDataTypeStr=single
  Product1 : Product  OutDataTypeStr=single
  Subtract{1..5} (5 alike) : Sum  Inputs=+-  OutDataTypeStr=single
  temp_u : Outport
  wires:
  Product1/1 -> Subtract5/2
  Product/1 -> Subtract5/1
  Subtract1/1 -> Product1/2
  Subtract4/1 -> Product1/1
  n1/1 -> Subtract3/2
  y2/1 -> Subtract4/1
  Subtract3/1 -> Product/2
  Subtract2/1 -> Product/1
  y1/1 -> Subtract4/2, Subtract3/1
  x1/1 -> Subtract1/1, Subtract2/2
  x2/1 -> Subtract2/1
  m1/1 -> Subtract1/2
  Subtract5/1 -> temp_u/1

== system TosAeb/FcwAebJa_Step_sys/ALG_AEBSystem/ALG_AEBController/AEB_LongTgtInfoCalc/LongObjCalcInfoCalc/AEBS_LongCollisionAssesApp/LongCollisionAvoidAssess/HighLvlBrkCalc/HighLvlBrkCalc4Brake/accelAvoidHighLvlBrkSituation1/accelAvoidLowLvlBrkCalc/Situation1 ==
  dxEgoBrake : Inport
  vx : Inport  Port=2
  tObjStop : Inport  Port=3
  subjVel : Inport  Port=4
  vxAbsObj : Inport  Port=5
  axAbsObj : Inport  Port=6
  function : TriggerPort  TriggerType=function-call  VariantControl=Variant
  Bus Creator : BusCreator  Inputs=6
  Bus Selector : BusSelector  OutputSignals=dxEgoBrake,vx,tObjStop
  Bus Selector1 : BusSelector  OutputSignals=axAbsObj,vx,dxEgoBrake
  Bus Selector2 : BusSelector  OutputSignals=subjVel,vxAbsObj,axAbsObj,dxEgoBrake
  Case1 : Subsystem  TreatAsAtomicUnit=on
  Case2 : Subsystem  TreatAsAtomicUnit=on
  If : If  IfExpression=u1
  Merge : Merge
  collisionFirstFlgCalc : Subsystem
  accelAvoidLowLvlBrk : Outport
  wires:
  If/1 -> Case1/Action
  Merge/1 -> accelAvoidLowLvlBrk/1
  Case1/1(accelAvoid) -> Merge/1
  Case2/1(accelAvoid) -> Merge/2
  If/2 -> [CASE2]
  [CASE2] -> Case2/Action
  tObjStop/1 -> Bus Creator/3  "tObjStop"
  vx/1 -> Bus Creator/2  "vx"
  subjVel/1 -> Bus Creator/4  "subjVel"
  dxEgoBrake/1 -> Bus Creator/1  "dxEgoBrake"
  axAbsObj/1 -> Bus Creator/6  "axAbsObj"
  vxAbsObj/1 -> Bus Creator/5  "vxAbsObj"
  Bus Creator/1 -> Bus Selector2/1, Bus Selector1/1, Bus Selector/1
  Bus Selector1/1 -> Case1/1(axAbsObj)  "<axAbsObj>"
  Bus Selector1/2 -> Case1/2(vx)  "<vx>"
  Bus Selector1/3 -> Case1/3(dxEgoBrake)  "<dxEgoBrake>"
  Bus Selector2/1 -> Case2/1(subjVel)  "<subjVel>"
  Bus Selector2/2 -> Case2/2(vxAbsObj)  "<vxAbsObj>"
  Bus Selector2/3 -> Case2/3(axAbsObj)  "<axAbsObj>"
  Bus Selector2/4 -> Case2/4(dxEgoBrake)  "<dxEgoBrake>"
  Bus Selector/1 -> collisionFirstFlgCalc/1(dxEgoBrake)  "<dxEgoBrake>"
  Bus Selector/2 -> collisionFirstFlgCalc/2(vx)  "<vx>"
  Bus Selector/3 -> collisionFirstFlgCalc/3(tObjStop)  "<tObjStop>"
  collisionFirstFlgCalc/1(collisionFirstFlg) -> If/1

== system TosAeb/FcwAebJa_Step_sys/ALG_AEBSystem/ALG_AEBController/AEB_LongTgtInfoCalc/LongObjCalcInfoCalc/AEBS_LongCollisionAssesApp/LongCollisionAvoidAssess/HighLvlBrkCalc/HighLvlBrkCalc4Brake/accelAvoidHighLvlBrkSituation1/accelAvoidLowLvlBrkCalc/Situation2 ==
  axAbsObj : Inport
  vx : Inport  Port=2
  dxEgoBrake : Inport  Port=3
  function : TriggerPort  TriggerType=function-call  VariantControl=Variant
  Divide : Product  Inputs=*/
  Gain : Gain  Gain=2
  Math Function : Math  Operator=square  SignedPower=on  SaturateOnIntegerOverflow=on
  Subtract : Sum  Inputs=+-
  accelAvoidLowLvlBrk : Outport
  wires:
  axAbsObj/1 -> Subtract/1
  vx/1 -> Math Function/1
  Math Function/1 -> Divide/1
  dxEgoBrake/1 -> Gain/1
  Gain/1 -> Divide/2
  Subtract/1 -> accelAvoidLowLvlBrk/1
  Divide/1 -> Subtract/2

== system TosAeb/FcwAebJa_Step_sys/ALG_AEBSystem/ALG_AEBController/AEB_LongTgtInfoCalc/LongObjCalcInfoCalc/AEBS_LongCollisionAssesApp/LongCollisionAvoidAssess/HighLvlBrkCalc/HighLvlBrkCalc4Brake/accelAvoidHighLvlBrkSituation1/accelAvoidLowLvlBrkCalc/Situation3 ==
  note: aEgo^2
      | aAvoid = ----------------------------------
      | 		vObj^2
      | 		---------  - 2(dRel - dScure)
      | 		aObj
  subjVel : Inport
  vxAbsObj : Inport  Port=2
  axAbsObj : Inport  Port=3
  dxEgoBrake : Inport  Port=4
  function : TriggerPort  TriggerType=function-call  VariantControl=Variant
  Divide : Product  Inputs=*/
  Divide1 : Product  Inputs=*/
  Gain : Gain  Gain=2
  Math Function : Math  Operator=square  SignedPower=on  SaturateOnIntegerOverflow=on
  Math Function1 : Math  Operator=square  SignedPower=on  SaturateOnIntegerOverflow=on
  Subtract : Sum  Inputs=+-
  accelAvoidLowLvlBrk : Outport
  wires:
  vxAbsObj/1 -> Math Function/1
  subjVel/1 -> Math Function1/1
  Math Function/1 -> Divide/1
  Gain/1 -> Subtract/2
  Divide1/1 -> accelAvoidLowLvlBrk/1
  Math Function1/1 -> Divide1/1
  Divide/1 -> Subtract/1
  axAbsObj/1 -> Divide/2
  dxEgoBrake/1 -> Gain/1
  Subtract/1 -> Divide1/2

== system TosAeb/FcwAebJa_Step_sys/ALG_AEBSystem/ALG_AEBController/AEB_LongTgtInfoCalc/LongObjCalcInfoCalc/AEBS_LongCollisionAssesApp/LongCollisionAvoidAssess/HighLvlBrkCalc/HighLvlBrkCalc4Brake/accelAvoidHighLvlBrkSituation1/accelAvoidLowLvlBrkCalc/Situation4 ==
  function : TriggerPort  TriggerType=function-call  VariantControl=Variant
  Constant : Constant  Value=CNNull  VectorParams1D=off
  accelAvoidLowLvlBrk : Outport
  wires:
  Constant/1 -> accelAvoidLowLvlBrk/1

== system TosAeb/FcwAebJa_Step_sys/ALG_AEBSystem/ALG_AEBController/AEB_LongTgtInfoCalc/LongObjCalcInfoCalc/AEBS_LongCollisionAssesApp/LongCollisionAvoidAssess/HighLvlBrkCalc/HighLvlBrkCalc4Brake/accelAvoidHighLvlBrkSituation1/accelAvoidLowLvlBrkCalc/accelAvoidLowLvlBrkLimit ==
  accelAvoidLowLvlBrkNoLimit : Inport
  Saturation : Saturate  UpperLimit=CNNull  LowerLimit=CNaccelAvoidMin
      settings: AttributesFormatString=|
      | UpperLimit:%<UpperLimit>
      | LowerLimit:%<LowerLimit>
  accelAvoidLowLvlBrk : Outport
  wires:
  accelAvoidLowLvlBrkNoLimit/1 -> Saturation/1
  Saturation/1 -> accelAvoidLowLvlBrk/1

== system TosAeb/FcwAebJa_Step_sys/ALG_AEBSystem/ALG_AEBController/AEB_LongTgtInfoCalc/LongObjCalcInfoCalc/AEBS_LongCollisionAssesApp/LongCollisionAvoidAssess/HighLvlBrkCalc/HighLvlBrkCalc4Brake/accelAvoidHighLvlBrkSituation1/accelAvoidLowLvlBrkCalc2/Situation1 ==
  dxEgoBrake : Inport
  vx : Inport  Port=2
  tObjStop : Inport  Port=3
  subjVel : Inport  Port=4
  vxAbsObj : Inport  Port=5
  axAbsObj : Inport  Port=6
  function : TriggerPort  TriggerType=function-call  VariantControl=Variant
  Bus Creator : BusCreator  Inputs=6
  Bus Selector : BusSelector  OutputSignals=dxEgoBrake,vxRelTargetObjCalc,tObjStop
  Bus Selector1 : BusSelector  OutputSignals=axAbsTargetObjCalc,vxRelTargetObjCalc,dxEgoBrake
  Bus Selector2 : BusSelector  OutputSignals=subjVel,vxAbsTargetObjCalc,axAbsTargetObjCalc,dxEgoBrake
  Case1 : Subsystem  TreatAsAtomicUnit=on
  Case2 : Subsystem  TreatAsAtomicUnit=on
  If : If  IfExpression=u1
  Merge : Merge
  collisionFirstFlgCalc : Subsystem
  accelAvoidLowLvlBrk : Outport
  wires:
  collisionFirstFlgCalc/1(collisionFirstFlg) -> If/1
  Bus Selector/3 -> collisionFirstFlgCalc/3(tObjStop)  "<tObjStop>"
  Bus Selector/2 -> collisionFirstFlgCalc/2(vx)  "<vxRelTargetObjCalc>"
  Bus Selector/1 -> collisionFirstFlgCalc/1(dxEgoBrake)  "<dxEgoBrake>"
  Bus Selector2/4 -> Case2/4(dxEgoBrake)  "<dxEgoBrake>"
  Bus Selector2/3 -> Case2/3(axAbsObj)  "<axAbsTargetObjCalc>"
  Bus Selector2/2 -> Case2/2(vxAbsObj)  "<vxAbsTargetObjCalc>"
  Bus Selector2/1 -> Case2/1(subjVel)  "<subjVel>"
  Bus Selector1/3 -> Case1/3(dxEgoBrake)  "<dxEgoBrake>"
  Bus Selector1/2 -> Case1/2(vx)  "<vxRelTargetObjCalc>"
  Bus Selector1/1 -> Case1/1(axAbsObj)  "<axAbsTargetObjCalc>"
  Bus Creator/1 -> Bus Selector/1, Bus Selector1/1, Bus Selector2/1
  vxAbsObj/1 -> Bus Creator/5  "vxAbsTargetObjCalc"
  axAbsObj/1 -> Bus Creator/6  "axAbsTargetObjCalc"
  dxEgoBrake/1 -> Bus Creator/1  "dxEgoBrake"
  subjVel/1 -> Bus Creator/4  "subjVel"
  vx/1 -> Bus Creator/2  "vxRelTargetObjCalc"
  tObjStop/1 -> Bus Creator/3  "tObjStop"
  [CASE2] -> Case2/Action
  If/2 -> [CASE2]
  Case2/1(accelAvoid) -> Merge/2
  Case1/1(accelAvoid) -> Merge/1
  Merge/1 -> accelAvoidLowLvlBrk/1
  If/1 -> Case1/Action

== system TosAeb/FcwAebJa_Step_sys/ALG_AEBSystem/ALG_AEBController/AEB_LongTgtInfoCalc/LongObjCalcInfoCalc/AEBS_LongCollisionAssesApp/LongCollisionAvoidAssess/HighLvlBrkCalc/HighLvlBrkCalc4Brake/accelAvoidHighLvlBrkSituation1/accelAvoidLowLvlBrkCalc2/Situation2 ==
  axAbsObj : Inport
  vx : Inport  Port=2
  dxEgoBrake : Inport  Port=3
  function : TriggerPort  TriggerType=function-call  VariantControl=Variant
  Divide : Product  Inputs=*/
  Gain : Gain  Gain=2
  Math Function : Math  Operator=square  SignedPower=on  SaturateOnIntegerOverflow=on
  Subtract : Sum  Inputs=+-
  accelAvoidLowLvlBrk : Outport
  wires:
  Divide/1 -> Subtract/2
  Subtract/1 -> accelAvoidLowLvlBrk/1
  Gain/1 -> Divide/2
  dxEgoBrake/1 -> Gain/1
  Math Function/1 -> Divide/1
  vx/1 -> Math Function/1
  axAbsObj/1 -> Subtract/1

== system TosAeb/FcwAebJa_Step_sys/ALG_AEBSystem/ALG_AEBController/AEB_LongTgtInfoCalc/LongObjCalcInfoCalc/AEBS_LongCollisionAssesApp/LongCollisionAvoidAssess/HighLvlBrkCalc/HighLvlBrkCalc4Brake/accelAvoidHighLvlBrkSituation1/accelAvoidLowLvlBrkCalc2/Situation3 ==
  note: aEgo^2
      | aAvoid = ----------------------------------
      | 		vObj^2
      | 		---------  - 2(dRel - dScure)
      | 		aObj
  subjVel : Inport
  vxAbsObj : Inport  Port=2
  axAbsObj : Inport  Port=3
  dxEgoBrake : Inport  Port=4
  function : TriggerPort  TriggerType=function-call  VariantControl=Variant
  Divide : Product  Inputs=*/
  Divide1 : Product  Inputs=*/
  Gain : Gain  Gain=2
  Math Function : Math  Operator=square  SignedPower=on  SaturateOnIntegerOverflow=on
  Math Function1 : Math  Operator=square  SignedPower=on  SaturateOnIntegerOverflow=on
  Subtract : Sum  Inputs=+-
  accelAvoidLowLvlBrk : Outport
  wires:
  Subtract/1 -> Divide1/2
  dxEgoBrake/1 -> Gain/1
  axAbsObj/1 -> Divide/2
  Divide/1 -> Subtract/1
  Math Function1/1 -> Divide1/1
  Divide1/1 -> accelAvoidLowLvlBrk/1
  Gain/1 -> Subtract/2
  Math Function/1 -> Divide/1
  subjVel/1 -> Math Function1/1
  vxAbsObj/1 -> Math Function/1

== system TosAeb/FcwAebJa_Step_sys/ALG_AEBSystem/ALG_AEBController/AEB_LongTgtInfoCalc/LongObjCalcInfoCalc/AEBS_LongCollisionAssesApp/LongCollisionAvoidAssess/HighLvlBrkCalc/HighLvlBrkCalc4Brake/accelAvoidHighLvlBrkSituation1/accelAvoidLowLvlBrkCalc2/Situation4 ==
  function : TriggerPort  TriggerType=function-call  VariantControl=Variant
  Constant : Constant  Value=CNaccelAvoidMax  VectorParams1D=off
  accelAvoidLowLvlBrk : Outport
  wires:
  Constant/1 -> accelAvoidLowLvlBrk/1

== system TosAeb/FcwAebJa_Step_sys/ALG_AEBSystem/ALG_AEBController/AEB_LongTgtInfoCalc/LongObjCalcInfoCalc/AEBS_LongCollisionAssesApp/LongCollisionAvoidAssess/HighLvlBrkCalc/HighLvlBrkCalc4Brake/accelAvoidHighLvlBrkSituation1/accelAvoidLowLvlBrkCalc2/accelAvoidLowLvlBrkLimit ==
  accelAvoidLowLvlBrkNoLimit : Inport
  Saturation : Saturate  UpperLimit=CNaccelAvoidMax  LowerLimit=CNaccelAvoidMin
      settings: AttributesFormatString=|
      | UpperLimit:%<UpperLimit>
      | LowerLimit:%<LowerLimit>
  accelAvoidLowLvlBrk : Outport
  wires:
  Saturation/1 -> accelAvoidLowLvlBrk/1
  accelAvoidLowLvlBrkNoLimit/1 -> Saturation/1

== system TosAeb/FcwAebJa_Step_sys/ALG_AEBSystem/ALG_AEBController/AEB_LongTgtInfoCalc/LongObjCalcInfoCalc/AEBS_LongCollisionAssesApp/LongCollisionAvoidAssess/HighLvlBrkCalc/HighLvlBrkCalc4Brake/accelAvoidHighLvlBrkSituation2/accelAvoidHighLvlBrkCalc/If Action Subsystem ==
  Action Port : ActionPort  ActionPortLabel=if(u1 <= 0)
  Constant : Constant  Value=CNPEBSAccelNegInf
  accelAvoidHighLvlBrk : Outport
  wires:
  Constant/1 -> accelAvoidHighLvlBrk/1  "CNPEBSAccelNegInf"

== system TosAeb/FcwAebJa_Step_sys/ALG_AEBSystem/ALG_AEBController/AEB_LongTgtInfoCalc/LongObjCalcInfoCalc/AEBS_LongCollisionAssesApp/LongCollisionAvoidAssess/HighLvlBrkCalc/HighLvlBrkCalc4Brake/accelAvoidHighLvlBrkSituation2/accelAvoidHighLvlBrkCalc/If Action Subsystem1 ==
  vx : Inport
  axAbsObj : Inport  Port=2
  vxAbsObj : Inport  Port=3
  subjVel : Inport  Port=4
  tObjStop : Inport  Port=5
  dxEgoBrake : Inport  Port=6
  Action Port : ActionPort  ActionPortLabel=else
  accelAvoidLowLvlBrkCalc : Subsystem  TreatAsAtomicUnit=on
  accelAvoidLowLvlBrkCalc1 : Subsystem  Commented=on  TreatAsAtomicUnit=on
  accelAvoidHighLvlBrk : Outport
  wires:
  vx/1 -> accelAvoidLowLvlBrkCalc/1(vx)
  axAbsObj/1 -> accelAvoidLowLvlBrkCalc/2(axAbsObj)
  vxAbsObj/1 -> accelAvoidLowLvlBrkCalc/3(vxAbsObj)
  subjVel/1 -> accelAvoidLowLvlBrkCalc/4(subjVel)
  tObjStop/1 -> accelAvoidLowLvlBrkCalc/5(tObjStop)
  dxEgoBrake/1 -> accelAvoidLowLvlBrkCalc/6(dxEgoBrake)
  accelAvoidLowLvlBrkCalc/1(accelAvoidLowLvlBrk) -> accelAvoidHighLvlBrk/1

== system TosAeb/FcwAebJa_Step_sys/ALG_AEBSystem/ALG_AEBController/AEB_LongTgtInfoCalc/LongObjCalcInfoCalc/AEBS_LongCollisionAssesApp/LongCollisionAvoidAssess/HighLvlBrkCalc/HighLvlBrkCalc4Brake/accelAvoidHighLvlBrkSituation2/accelAvoidHighLvlBrkCalc/MovingSateCalcAfterReactionTime ==
  note: Both Ego and Obj keep moving after driver reaction time
  dxFrontBumper : Inport
  vx : Inport  Port=2
  tReaction : Inport  Port=3
  ax : Inport  Port=4
  subjLongAccel : Inport  Port=5
  subjVel : Inport  Port=6
  Add : Sum  Inputs=+++
  Add1 : Sum
  Add2 : Sum
  Add3 : Sum
  Constant : Constant  Value=CNNull
  Gain : Gain  Gain=0.5
  Math Function : Math  Operator=square  SignedPower=on  SaturateOnIntegerOverflow=on
  MinMax : MinMax  Function=max  Inputs=2
  Product{,1..3} (4 alike) : Product
  dxFrontBumperPredict : Outport
  vxPredict : Outport  Port=2
  vxAbsObjPredict : Outport  Port=3
  subjVelPredict : Outport  Port=4
  wires:
  MinMax/1 -> vxAbsObjPredict/1
  Constant/1 -> MinMax/2
  Product2/1 -> Add1/1
  tReaction/1 -> Product3/1, Product2/1, Math Function/1, Product1/2
  ax/1 -> Product2/2, Product/2
  Add1/1 -> Add3/1, vxPredict/1  "vxPredict"
  subjVel/1 -> Add2/2
  Product/1 -> Gain/1
  vx/1 -> Product1/1, Add1/2
  Gain/1 -> Add/3
  Product1/1 -> Add/2
  Product3/1 -> Add2/1
  Add/1 -> dxFrontBumperPredict/1  "dxFrontBumperPredict"
  Add2/1 -> Add3/2, subjVelPredict/1  "subjVelPredict"
  subjLongAccel/1 -> Product3/2
  Math Function/1 -> Product/1
  Add3/1 -> MinMax/1
  dxFrontBumper/1 -> Add/1

== system TosAeb/FcwAebJa_Step_sys/ALG_AEBSystem/ALG_AEBController/AEB_LongTgtInfoCalc/LongObjCalcInfoCalc/AEBS_LongCollisionAssesApp/LongCollisionAvoidAssess/HighLvlBrkCalc/HighLvlBrkCalc4Brake/accelAvoidHighLvlBrkSituation2/accelAvoidHighLvlBrkCalc/tObjStopCalc ==
  accelRel : Inport
  velRel : Inport  Port=2
  StopTimeCalc : Subsystem  TreatAsAtomicUnit=on
  StopTimeFlow : Stateflow chart  TreatAsAtomicUnit=on
  tStop : Outport
  wires:
  velRel/1 -> StopTimeFlow/2(velRel), StopTimeCalc/1(velRel)  "velRel"
  accelRel/1 -> StopTimeFlow/1(accelRel), StopTimeCalc/2(accelRel)  "accelRel"
  StopTimeFlow/1(tStop) -> tStop/1  "tStop"
  [tStopIn] -> StopTimeFlow/3(tStopIn)
  StopTimeCalc/1(tStop) -> [tStopIn]  "tStopIn"
  StopTimeFlow/2(tStopFuncCalc()) -> StopTimeCalc/Trigger

== system TosAeb/FcwAebJa_Step_sys/ALG_AEBSystem/ALG_AEBController/AEB_LongTgtInfoCalc/LongObjCalcInfoCalc/AEBS_LongCollisionAssesApp/LongCollisionAvoidAssess/HighLvlBrkCalc/HighLvlBrkCalc4Brake/accelAvoidHighLvlBrkSituation3/If Action Subsystem1/CollisionOcurredCase ==
  Action Port : ActionPort  ActionPortLabel=if(u1)
  Constant : Constant  Value=CNaccelAvoidMin
  accelAvoidHighLvlBrk : Outport
  wires:
  Constant/1 -> accelAvoidHighLvlBrk/1  "CNPEBSAccelNegInf"
